simulink model "Motor"  (R2018a)
settings: Solver=ode23tb  StartTime=0.0  StopTime=0.1  MaxStep=10e-6  RelTol=1e-3
undefined symbols (not defined in the file or in its folder): sigbuilder_block
libraries: powerlib x1
settings Model: Creator=Ilian  LastModifiedBy=Ilian
settings SolverCC: InitialStep=10e-8

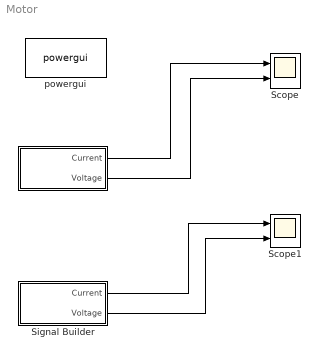
== system Motor ==
  " " : Subsystem  Tag=STV Subsys  LoadFcn=sigbuilder_block('load');  StartFcn=sigbuilder_block('start');  StopFcn=sigbuilder_block('stop');  ClipboardFcn=sigbuilder_block('clipboard');  mask(Sigbuilder block){tuvar=[0:10]}
      mask help: Simulink:masks:SigbuilderBlock_MD
      mask init:
      | if ~strcmp(get_param(bdroot(gcbh),'SimulationStatus'),'stopped') tuvar = sigbuilder_block('maskInit'); end
      settings: CopyFcn=sigbuilder_block('copy');  DeleteFcn=sigbuilder_block('delete');  ModelCloseFcn=sigbuilder_block('modelClose');  PreSaveFcn=sigbuilder_block('preSave');  PauseFcn=sigbuilder_block('pause');  ContinueFcn=sigbuilder_block('continue');  NameChangeFcn=sigbuilder_block('namechange');  OpenFcn=sigbuilder_block('open',[6 36 708 710.25 ]);  CloseFcn=sigbuilder_block('close');
      mask Display: plot(0, 0, 100, 100,[2, 2, 32, 32, 2], [68, 8, 8, 68, 68],[32, 2], [38, 38], [32, 19, 2],[53, 60, 44], [32, 17, 17, 2],[16, 16, 31, 31]);txt = getActiveGroup(gcbh);text(2, 100, txt,'verticalAlignment', 'top');
  Scope : Scope  NumInputPorts=2
  Scope1 : Scope  NumInputPorts=2
  Signal Builder : Subsystem  Tag=STV Subsys  LoadFcn=sigbuilder_block('load');  StartFcn=sigbuilder_block('start');  StopFcn=sigbuilder_block('stop');  ClipboardFcn=sigbuilder_block('clipboard');  mask(Sigbuilder block){tuvar=[0:10]}
      mask help: Simulink:masks:SigbuilderBlock_MD
      mask init:
      | if ~strcmp(get_param(bdroot(gcbh),'SimulationStatus'),'stopped') tuvar = sigbuilder_block('maskInit'); end
      settings: CopyFcn=sigbuilder_block('copy');  DeleteFcn=sigbuilder_block('delete');  ModelCloseFcn=sigbuilder_block('modelClose');  PreSaveFcn=sigbuilder_block('preSave');  PauseFcn=sigbuilder_block('pause');  ContinueFcn=sigbuilder_block('continue');  NameChangeFcn=sigbuilder_block('namechange');  OpenFcn=sigbuilder_block('open',[254.625 4.875 700.5 477.75 ]);  CloseFcn=sigbuilder_block('close');
      mask Display: plot(0, 0, 100, 100,[2, 2, 32, 32, 2], [68, 8, 8, 68, 68],[32, 2], [38, 38], [32, 19, 2],[53, 60, 44], [32, 17, 17, 2],[16, 16, 31, 31]);txt = getActiveGroup(gcbh);text(2, 100, txt,'verticalAlignment', 'top');
  powergui : powerlib/powergui  Priority=1  SimulationMode=Continuous  SampleTime=50e-6  frequency=60  Iterations=50  frequencyindice=60  Pbase=100e6  ErrMax=1e-4  UnitsV=kV  UnitsW=MW  buscounter=0  FunctionMessages=off  echomessages=off  EnableUseOfTLC=off  CurrentSourceSwitches=off  DisableSnubberDevices=off  DisableRonSwitches=off  DisableVfSwitches=off  DisplayEquations=off  SolverType=Tustin/Backward Euler (TBE)  Interpol=off  ExternalGateDelay=off  methode=off  Ts=100  x0status=blocks  SPID=on  SwTol=0  HookPort=off  RestoreLinks=warning  ResistiveCurrentMeasurement=off  Frange=[0:2:500]  Ylog=off  Xlog=on  ShowGrid=off  save=off  variable=ZData  structure=RPM700  ZoomFFT=off  StartTime=0.0  cycles=5  DisplayStyle=1  fundamental=50  FreqAxis=off  MaxFrequency=1000  frequencyindicesteady=1  RmsSteady=1  display=off
  wires:
  " "/1(Current) -> Scope/1
  " "/2(Voltage) -> Scope/2
  Signal Builder/1(Current) -> Scope1/1
  Signal Builder/2(Voltage) -> Scope1/2

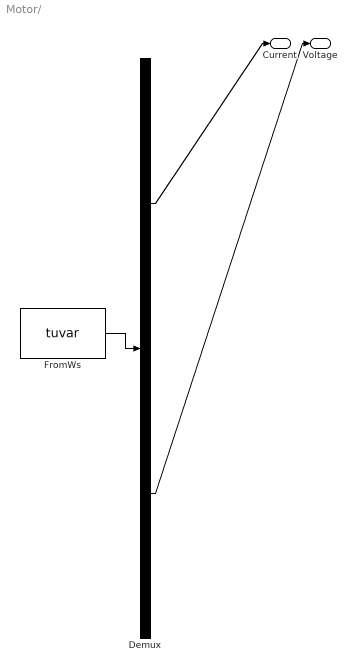
== system Motor/  ==
  Demux : Demux  Tag=STV Demux  Outputs=2
  FromWs : FromWorkspace  Tag=STV FromWs  VariableName=tuvar  SampleTime=0
      SigBuilderData=|
      | common.afterFinalStr = 'Extrapolate'
      | common.sampleTime = 0
      | dataSet(1).timeRange = [0 0.0999992]
      | dataSet(1).name = '700 RPM'
      | dataSet(2).timeRange = [0 0.0999992]
      | dataSet(2).name = '1400 RPM1'
      | sbobj.Groups(1).Name = '700 RPM'
      | sbobj.Groups(1).Signals(1).Name = 'Current'
      | sbobj.Groups(1).Signals(1).XData = [0 8.00000000002e-07 1.6e-06 2.4e-06 3.2e-06 4e-06 4.80000000001e-06 5.6e-06 6.4e-06 7.20000000001e-06 8e-06 8.8e-06 ... (125000 elements, 1x125000)]
      | sbobj.Groups(1).Signals(1).YData = [-1.7 -2.1 -1.9 -1.9 -1.9 -1.9 -1.9 -1.9 -2.1 -1.9 -2.1 -2.1 ... (125000 elements, 1x125000)]
      | sbobj.Groups(1).Signals(2).Name = 'Voltage'
      | sbobj.Groups(1).Signals(2).XData = [0 8.00000000002e-07 1.6e-06 2.4e-06 3.2e-06 4e-06 4.80000000001e-06 5.6e-06 6.4e-06 7.20000000001e-06 8e-06 8.8e-06 ... (125000 elements, 1x125000)]
      | sbobj.Groups(1).Signals(2).YData = [20 20 20 20 20 20 20 20 20 20 20 20 ... (125000 elements, 1x125000)]
      | sbobj.Groups(1).TimeRange = [0 0.0999992]
      | sbobj.Groups(2).Name = '1400 RPM1'
      | sbobj.Groups(2).Signals(1).Name = 'Current'
      | sbobj.Groups(2).Signals(1).XData = [0 8.00000000002e-07 1.6e-06 2.4e-06 3.2e-06 4e-06 4.80000000001e-06 5.6e-06 6.4e-06 7.20000000001e-06 8e-06 8.8e-06 ... (125000 elements, 1x125000)]
      | sbobj.Groups(2).Signals(1).YData = [-1.7 -2.1 -1.9 -1.9 -1.9 -1.9 -1.9 -1.9 -2.1 -1.9 -2.1 -2.1 ... (125000 elements, 1x125000)]
      | sbobj.Groups(2).Signals(2).Name = 'Voltage'
      | sbobj.Groups(2).Signals(2).XData = [0 8.00000000002e-07 1.6e-06 2.4e-06 3.2e-06 4e-06 4.80000000001e-06 5.6e-06 6.4e-06 7.20000000001e-06 8e-06 8.8e-06 ... (125000 elements, 1x125000)]
      | sbobj.Groups(2).Signals(2).YData = [20 20 20 20 20 20 20 20 20 20 20 20 ... (125000 elements, 1x125000)]
      | sbobj.Groups(2).TimeRange = [0 0.0999992]
  Current : Outport  Tag=STV Outport
  Voltage : Outport  Tag=STV Outport  Port=2
  wires:
  FromWs/1 -> Demux/1
  Demux/1 -> Current/1
  Demux/2 -> Voltage/1

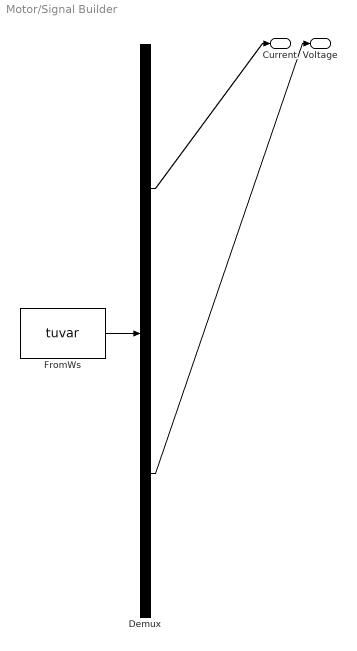
== system Motor/Signal Builder ==
  Demux : Demux  Tag=STV Demux  Outputs=2
  FromWs : FromWorkspace  Tag=STV FromWs  VariableName=tuvar  SampleTime=0
      SigBuilderData=|
      | common.afterFinalStr = 'Extrapolate'
      | common.sampleTime = 0
      | dataSet.timeRange = [0 0.1]
      | dataSet.name = '1400 RPM'
      | sbobj.Groups.Name = '1400 RPM'
      | sbobj.Groups.Signals(1).Name = 'Current'
      | sbobj.Groups.Signals(1).XData = [0 8.00000000002e-07 1.6e-06 2.4e-06 3.2e-06 4e-06 4.80000000001e-06 5.6e-06 6.4e-06 7.20000000001e-06 8e-06 8.8e-06 ... (125001 elements, 1x125001)]
      | sbobj.Groups.Signals(1).YData = [-2.7 -2.5 -2.5 -2.5 -2.7 -2.5 -2.5 -2.5 -2.7 -2.7 -2.7 -2.5 ... (125001 elements, 1x125001)]
      | sbobj.Groups.Signals(2).Name = 'Voltage'
      | sbobj.Groups.Signals(2).XData = [0 8.00000000002e-07 1.6e-06 2.4e-06 3.2e-06 4e-06 4.80000000001e-06 5.6e-06 6.4e-06 7.20000000001e-06 8e-06 8.8e-06 ... (125000 elements, 1x125000)]
      | sbobj.Groups.Signals(2).YData = [20 20 60 -20 20 -20 20 20 20 20 20 20 ... (125000 elements, 1x125000)]
      | sbobj.Groups.TimeRange = [0 0.1]
  Current : Outport  Tag=STV Outport
  Voltage : Outport  Tag=STV Outport  Port=2
  wires:
  FromWs/1 -> Demux/1
  Demux/1 -> Current/1
  Demux/2 -> Voltage/1
